simulink model "MX_Gateway"  (Simulink 9.0)
settings: Solver=FixedStepDiscrete  StartTime=0.0  StopTime=100  FixedStep=0.01  RelTol=1e-3
values from neighbouring files (symbols this model uses):
  ACTIVE = Bobcat.Parameter; CreateDefine( ACTIVE                         ,1                          ,'Define' ,'defines.h' )    [defines.m]
  ACTIVE_MODE_ONE = Bobcat.Parameter; CreateDefine( ACTIVE_MODE_ONE                ,19                         ,'Define' ,'defines.h' )    [defines.m]
  AUTHENTICATED = Bobcat.Parameter; CreateDefine( AUTHENTICATED                  ,1                          ,'Define' ,'defines.h' )    [defines.m]
  AUX_EMEA_CONTROLS = Bobcat.Parameter; CreateDefine( AUX_EMEA_CONTROLS  ,1   ,'Define' ,'defines.h' )    [enums.m]
  AUX_FLOW_HIGH = Bobcat.Parameter; CreateDefine( AUX_FLOW_HIGH           ,4  ,'Define' ,'defines.h' )    [enums.m]
  AUX_FLOW_INACTIVE = Bobcat.Parameter; CreateDefine( AUX_FLOW_INACTIVE       ,0  ,'Define' ,'defines.h' )    [enums.m]
  AUX_FLOW_LOW = Bobcat.Parameter; CreateDefine( AUX_FLOW_LOW            ,1  ,'Define' ,'defines.h' )    [enums.m]
  AUX_FLOW_MEDIUM = Bobcat.Parameter; CreateDefine( AUX_FLOW_MEDIUM         ,2  ,'Define' ,'defines.h' )    [enums.m]
  AUX_FLOW_STANDARD = Bobcat.Parameter; CreateDefine( AUX_FLOW_STANDARD       ,3  ,'Define' ,'defines.h' )    [enums.m]
  AUX_NON_FUNCTIONAL = Bobcat.Parameter; CreateDefine( AUX_NON_FUNCTIONAL ,255 ,'Define' ,'defines.h' )    [enums.m]
  BASE_ACTIVE = Bobcat.Parameter; CreateDefine( BASE_ACTIVE                    ,253                        ,'Define' ,'defines.h' )    [defines.m]
  BASE_DETENT = Bobcat.Parameter; CreateDefine( BASE_DETENT                    ,1                          ,'Define' ,'defines.h' )    [defines.m]
  BEEP_NONE = Bobcat.Parameter; CreateDefine( BEEP_NONE                      ,255                        ,'Define' ,'defines.h' )    [defines.m]
  CD_AIR_FILTER_DEBOUNCE_NORMAL = Bobcat.Parameter; CreateAliasCD( CD_AIR_FILTER_DEBOUNCE_NORMAL                        ,uint16(500)                ,'ConstantDataPointer' ,'CONSTANT_DATA' ,'AIR_FILTER_DEBOUNCE_NORMAL')    [cdata.m]    (also in hc12_cdata.m; m4_cdata.m)
  CD_AIR_FILTER_DEBOUNCE_NOT_CONNECTED = Bobcat.Parameter; CreateAliasCD( CD_AIR_FILTER_DEBOUNCE_NOT_CONNECTED                 ,uint16(3000)               ,'ConstantDataPointer' ,'CONSTANT_DATA' ,'AIR_FILTER_DEBOUNCE_NOT_CONNECTED')    [cdata.m]    (also in hc12_cdata.m; m4_cdata.m)
  CD_AIR_FILTER_DEBOUNCE_PLUGGED = Bobcat.Parameter; CreateAliasCD( CD_AIR_FILTER_DEBOUNCE_PLUGGED                       ,uint16(3000)               ,'ConstantDataPointer' ,'CONSTANT_DATA' ,'AIR_FILTER_DEBOUNCE_PLUGGED')    [cdata.m]    (also in hc12_cdata.m; m4_cdata.m)
  CD_AIR_FILTER_FUNCTION = Bobcat.Parameter; CreateAliasCD( CD_AIR_FILTER_FUNCTION                               ,uint8(255)                 ,'ConstantDataPointer' ,'CONSTANT_DATA' ,'AIR_FILTER_FUNCTION')    [cdata.m]    (also in hc12_cdata.m; m4_cdata.m)
  CD_AIR_FILTER_PLUGGED_WEIGHT = Bobcat.Parameter; CreateAliasCD( CD_AIR_FILTER_PLUGGED_WEIGHT                         ,uint8(2)                   ,'ConstantDataPointer' ,'CONSTANT_DATA' ,'AIR_FILTER_PLUGGED_WEIGHT')    [cdata.m]    (also in hc12_cdata.m; m4_cdata.m)
  CD_AIR_FILTER_UNPLUGGED_WEIGHT = Bobcat.Parameter; CreateAliasCD( CD_AIR_FILTER_UNPLUGGED_WEIGHT                       ,uint8(1)                   ,'ConstantDataPointer' ,'CONSTANT_DATA' ,'AIR_FILTER_UNPLUGGED_WEIGHT')    [cdata.m]    (also in hc12_cdata.m; m4_cdata.m)
  CD_AUTOIDLE_ARMBAR_DELAY = Bobcat.Parameter; CreateAliasCD( CD_AUTOIDLE_ARMBAR_DELAY                             ,uint8(2)                   ,'ConstantDataPointer' ,'CONSTANT_DATA' ,'AUTOIDLE_ARMBAR_DELAY')    [cdata.m]    (also in hc12_cdata.m; m4_cdata.m)
  CD_AUTOIDLE_FUNCTION = Bobcat.Parameter; CreateAliasCD( CD_AUTOIDLE_FUNCTION                                 ,uint8(255)                 ,'ConstantDataPointer' ,'CONSTANT_DATA' ,'AUTOIDLE_FUNCTION')    [cdata.m]    (also in hc12_cdata.m; m4_cdata.m)
  CD_AUTOIDLE_IDLE_DELAY = Bobcat.Parameter; CreateAliasCD( CD_AUTOIDLE_IDLE_DELAY                               ,uint8(40)                  ,'ConstantDataPointer' ,'CONSTANT_DATA' ,'AUTOIDLE_IDLE_DELAY')    [cdata.m]    (also in hc12_cdata.m; m4_cdata.m)
  CD_AUTOIDLE_LOW_IDLE_START = Bobcat.Parameter; CreateAliasCD( CD_AUTOIDLE_LOW_IDLE_START                           ,uint8(255)                 ,'ConstantDataPointer' ,'CONSTANT_DATA' ,'AUTOIDLE_LOW_IDLE_START')    [cdata.m]    (also in hc12_cdata.m; m4_cdata.m)
  CD_AUTOIDLE_PRESSURE_OFF = Bobcat.Parameter; CreateAliasCD( CD_AUTOIDLE_PRESSURE_OFF                             ,uint16(300)                ,'ConstantDataPointer' ,'CONSTANT_DATA' ,'AUTOIDLE_PRESSURE_OFF')    [cdata.m]    (also in hc12_cdata.m; m4_cdata.m)
  CD_AUTOIDLE_PRESSURE_ON = Bobcat.Parameter; CreateAliasCD( CD_AUTOIDLE_PRESSURE_ON                              ,uint16(270)                ,'ConstantDataPointer' ,'CONSTANT_DATA' ,'AUTOIDLE_PRESSURE_ON')    [cdata.m]    (also in hc12_cdata.m; m4_cdata.m)
  CD_AUTOSHIFT_FUNCTION = Bobcat.Parameter; CreateAliasCD( CD_AUTOSHIFT_FUNCTION                                ,uint8(255)                 ,'ConstantDataPointer' ,'CONSTANT_DATA' ,'AUTOSHIFT_FUNCTION')    [cdata.m]    (also in hc12_cdata.m; m4_cdata.m)
  CD_AUTOSHIFT_PHIGH1 = Bobcat.Parameter; CreateAliasCD( CD_AUTOSHIFT_PHIGH1                                  ,uint16(205)                ,'ConstantDataPointer' ,'CONSTANT_DATA' ,'AUTOSHIFT_PHIGH1')    [cdata.m]    (also in hc12_cdata.m; m4_cdata.m)
  CD_AUTOSHIFT_PHIGH2 = Bobcat.Parameter; CreateAliasCD( CD_AUTOSHIFT_PHIGH2                                  ,uint16(193)                ,'ConstantDataPointer' ,'CONSTANT_DATA' ,'AUTOSHIFT_PHIGH2')    [cdata.m]    (also in hc12_cdata.m; m4_cdata.m)
  CD_AUTOSHIFT_PHIGH_TEMP1 = Bobcat.Parameter; CreateAliasCD( CD_AUTOSHIFT_PHIGH_TEMP1                             ,int16(70)                  ,'ConstantDataPointer' ,'CONSTANT_DATA' ,'AUTOSHIFT_PHIGH_TEMP1')    [cdata.m]    (also in hc12_cdata.m; m4_cdata.m)
  CD_AUTOSHIFT_PHIGH_TEMP2 = Bobcat.Parameter; CreateAliasCD( CD_AUTOSHIFT_PHIGH_TEMP2                             ,int16(160)                 ,'ConstantDataPointer' ,'CONSTANT_DATA' ,'AUTOSHIFT_PHIGH_TEMP2')    [cdata.m]    (also in hc12_cdata.m; m4_cdata.m)
  CD_AUTOSHIFT_PLOW1 = Bobcat.Parameter; CreateAliasCD( CD_AUTOSHIFT_PLOW1                                   ,uint16(130)                ,'ConstantDataPointer' ,'CONSTANT_DATA' ,'AUTOSHIFT_PLOW1')    [cdata.m]    (also in hc12_cdata.m; m4_cdata.m)
  CD_AUTOSHIFT_PLOW2 = Bobcat.Parameter; CreateAliasCD( CD_AUTOSHIFT_PLOW2                                   ,uint16(100)                ,'ConstantDataPointer' ,'CONSTANT_DATA' ,'AUTOSHIFT_PLOW2')    [cdata.m]    (also in hc12_cdata.m; m4_cdata.m)
  CD_AUTOSHIFT_PLOW_TEMP1 = Bobcat.Parameter; CreateAliasCD( CD_AUTOSHIFT_PLOW_TEMP1                              ,int16(20)                  ,'ConstantDataPointer' ,'CONSTANT_DATA' ,'AUTOSHIFT_PLOW_TEMP1')    [cdata.m]    (also in hc12_cdata.m; m4_cdata.m)
  CD_AUTOSHIFT_PLOW_TEMP2 = Bobcat.Parameter; CreateAliasCD( CD_AUTOSHIFT_PLOW_TEMP2                              ,int16(80)                  ,'ConstantDataPointer' ,'CONSTANT_DATA' ,'AUTOSHIFT_PLOW_TEMP2')    [cdata.m]    (also in hc12_cdata.m; m4_cdata.m)
  CD_AUTOSHIFT_TRAVEL_DELAY = Bobcat.Parameter; CreateAliasCD( CD_AUTOSHIFT_TRAVEL_DELAY                            ,uint8(32)                  ,'ConstantDataPointer' ,'CONSTANT_DATA' ,'AUTOSHIFT_TRAVEL_DELAY')    [cdata.m]    (also in hc12_cdata.m; m4_cdata.m)
  CD_AUX2_CRACKPOINT_CURRENT = Bobcat.Parameter; CreateAliasCD( CD_AUX2_CRACKPOINT_CURRENT                           ,fi(0,0,16,12,'hex','0c28') ,'ConstantDataPointer' ,'CONSTANT_DATA' ,'AUX2_CRACKPOINT_CURRENT')    [cdata.m]    (also in hc12_cdata.m; m4_cdata.m)
  CD_AUX2_FLOW_LOW_FUNCTION = Bobcat.Parameter; CreateAliasCD( CD_AUX2_FLOW_LOW_FUNCTION                            ,uint8(255)                 ,'ConstantDataPointer' ,'CONSTANT_DATA' ,'AUX2_FLOW_LOW_FUNCTION')    [cdata.m]    (also in hc12_cdata.m; m4_cdata.m)
  CD_AUX2_FLOW_MED_FUNCTION = Bobcat.Parameter; CreateAliasCD( CD_AUX2_FLOW_MED_FUNCTION                            ,uint8(255)                 ,'ConstantDataPointer' ,'CONSTANT_DATA' ,'AUX2_FLOW_MED_FUNCTION')    [cdata.m]    (also in hc12_cdata.m; m4_cdata.m)
  CD_AUX2_HI_FLOW_PERCENT = Bobcat.Parameter; CreateAliasCD( CD_AUX2_HI_FLOW_PERCENT                              ,fi(0,0,16,16,'hex','ffff') ,'ConstantDataPointer' ,'CONSTANT_DATA' ,'AUX2_HI_FLOW_PERCENT')    [cdata.m]    (also in hc12_cdata.m; m4_cdata.m)
  CD_AUX2_LOW_FLOW_PERCENT = Bobcat.Parameter; CreateAliasCD( CD_AUX2_LOW_FLOW_PERCENT                             ,fi(0,0,16,16,'hex','7333') ,'ConstantDataPointer' ,'CONSTANT_DATA' ,'AUX2_LOW_FLOW_PERCENT')    [cdata.m]    (also in hc12_cdata.m; m4_cdata.m)
  CD_AUX2_MAX_FLOW_CURRENT = Bobcat.Parameter; CreateAliasCD( CD_AUX2_MAX_FLOW_CURRENT                             ,fi(0,0,16,12,'hex','14cc') ,'ConstantDataPointer' ,'CONSTANT_DATA' ,'AUX2_MAX_FLOW_CURRENT')    [cdata.m]    (also in hc12_cdata.m; m4_cdata.m)
  CD_AUX2_MED_FLOW_PERCENT = Bobcat.Parameter; CreateAliasCD( CD_AUX2_MED_FLOW_PERCENT                             ,fi(0,0,16,16,'hex','9999') ,'ConstantDataPointer' ,'CONSTANT_DATA' ,'AUX2_MED_FLOW_PERCENT')    [cdata.m]    (also in hc12_cdata.m; m4_cdata.m)
  CD_AUX_CRACKPOINT_CURRENT = Bobcat.Parameter; CreateAliasCD( CD_AUX_CRACKPOINT_CURRENT                            ,fi(0,0,16,12,'hex','0000') ,'ConstantDataPointer' ,'CONSTANT_DATA' ,'AUX_CRACKPOINT_CURRENT')    [cdata.m]    (also in hc12_cdata.m; m4_cdata.m)
  CD_AUX_DETENT_FLASH_TIME = Bobcat.Parameter; CreateAliasCD( CD_AUX_DETENT_FLASH_TIME                             ,uint16(72)                 ,'ConstantDataPointer' ,'CONSTANT_DATA' ,'AUX_DETENT_FLASH_TIME')    [cdata.m]    (also in hc12_cdata.m; m4_cdata.m)
  CD_AUX_DETENT_HOLD_TIME = Bobcat.Parameter; CreateAliasCD( CD_AUX_DETENT_HOLD_TIME                              ,uint16(100)                ,'ConstantDataPointer' ,'CONSTANT_DATA' ,'AUX_DETENT_HOLD_TIME')    [cdata.m]    (also in hc12_cdata.m; m4_cdata.m)
  CD_AUX_FULL_ON_AUX1 = Bobcat.Parameter; CreateAliasCD( CD_AUX_FULL_ON_AUX1                                  ,fi(0,0,16,12,'hex','10cd') ,'ConstantDataPointer' ,'CONSTANT_DATA' ,'AUX_FULL_ON_AUX1')    [cdata.m]    (also in hc12_cdata.m; m4_cdata.m)
  CD_AUX_FULL_ON_AUX2 = Bobcat.Parameter; CreateAliasCD( CD_AUX_FULL_ON_AUX2                                  ,fi(0,0,16,12,'hex','139d') ,'ConstantDataPointer' ,'CONSTANT_DATA' ,'AUX_FULL_ON_AUX2')    [cdata.m]    (also in hc12_cdata.m; m4_cdata.m)
  CD_AUX_FULL_ON_AUX3 = Bobcat.Parameter; CreateAliasCD( CD_AUX_FULL_ON_AUX3                                  ,fi(0,0,16,12,'hex','17d7') ,'ConstantDataPointer' ,'CONSTANT_DATA' ,'AUX_FULL_ON_AUX3')    [cdata.m]    (also in hc12_cdata.m; m4_cdata.m)
  CD_AUX_FUNCTION = Bobcat.Parameter; CreateAliasCD( CD_AUX_FUNCTION                                      ,uint8(0)                   ,'ConstantDataPointer' ,'CONSTANT_DATA' ,'AUX_FUNCTION')    [cdata.m]    (also in hc12_cdata.m; m4_cdata.m)
  CD_AUX_RATE_LIMIT_CRACKPOINT = Bobcat.Parameter; CreateAliasCD( CD_AUX_RATE_LIMIT_CRACKPOINT                         ,fi(0,0,16,12,'hex','0800') ,'ConstantDataPointer' ,'CONSTANT_DATA' ,'AUX_RATE_LIMIT_CRACKPOINT')    [cdata.m]    (also in hc12_cdata.m; m4_cdata.m)
  CD_AUX_RATE_LIMIT_CRACKPOINT_RATE = Bobcat.Parameter; CreateAliasCD( CD_AUX_RATE_LIMIT_CRACKPOINT_RATE                    ,fi(0,1,32,12,'int',102400) ,'ConstantDataPointer' ,'CONSTANT_DATA' ,'AUX_RATE_LIMIT_CRACKPOINT_RATE')    [cdata.m]    (also in hc12_cdata.m; m4_cdata.m)
  CD_AUX_RATE_LIMIT_FUNCTION = Bobcat.Parameter; CreateAliasCD( CD_AUX_RATE_LIMIT_FUNCTION                           ,uint8(255)                 ,'ConstantDataPointer' ,'CONSTANT_DATA' ,'AUX_RATE_LIMIT_FUNCTION')    [cdata.m]    (also in hc12_cdata.m; m4_cdata.m)
  CD_AUX_RATE_LIMIT_MAX_ENG_SPD = Bobcat.Parameter; CreateAliasCD( CD_AUX_RATE_LIMIT_MAX_ENG_SPD                        ,uint16(1600)               ,'ConstantDataPointer' ,'CONSTANT_DATA' ,'AUX_RATE_LIMIT_MAX_ENG_SPD')    [cdata.m]    (also in hc12_cdata.m; m4_cdata.m)
  CD_AUX_RATE_LIMIT_MIN_ENG_SPD = Bobcat.Parameter; CreateAliasCD( CD_AUX_RATE_LIMIT_MIN_ENG_SPD                        ,uint16(100)                ,'ConstantDataPointer' ,'CONSTANT_DATA' ,'AUX_RATE_LIMIT_MIN_ENG_SPD')    [cdata.m]    (also in hc12_cdata.m; m4_cdata.m)
  CD_AUX_RATE_LIMIT_NORMAL_RATE = Bobcat.Parameter; CreateAliasCD( CD_AUX_RATE_LIMIT_NORMAL_RATE                        ,fi(0,1,32,12,'int',2048)   ,'ConstantDataPointer' ,'CONSTANT_DATA' ,'AUX_RATE_LIMIT_NORMAL_RATE')    [cdata.m]    (also in hc12_cdata.m; m4_cdata.m)
  CD_AUXPOT_CAL_DEADBAND_HIGH = Bobcat.Parameter; CreateAliasCD( CD_AUXPOT_CAL_DEADBAND_HIGH                          ,uint16(574)                ,'ConstantDataPointer' ,'CONSTANT_DATA' ,'AUXPOT_CAL_DEADBAND_HIGH')    [cdata.m]    (also in hc12_cdata.m; m4_cdata.m)
  CD_AUXPOT_CAL_DEADBAND_LOW = Bobcat.Parameter; CreateAliasCD( CD_AUXPOT_CAL_DEADBAND_LOW                           ,uint16(424)                ,'ConstantDataPointer' ,'CONSTANT_DATA' ,'AUXPOT_CAL_DEADBAND_LOW')    [cdata.m]    (also in hc12_cdata.m; m4_cdata.m)
  CD_AUXPOT_CENTER_RATIO = Bobcat.Parameter; CreateAliasCD( CD_AUXPOT_CENTER_RATIO                               ,uint16(500)                ,'ConstantDataPointer' ,'CONSTANT_DATA' ,'AUXPOT_CENTER_RATIO')    [cdata.m]    (also in hc12_cdata.m; m4_cdata.m)
  CD_AUXPOT_DEADBAND = Bobcat.Parameter; CreateAliasCD( CD_AUXPOT_DEADBAND                                   ,uint8(38)                  ,'ConstantDataPointer' ,'CONSTANT_DATA' ,'AUXPOT_DEADBAND')    [cdata.m]    (also in hc12_cdata.m; m4_cdata.m)
  CD_AUXPOT_FUNCTION = Bobcat.Parameter; CreateAliasCD( CD_AUXPOT_FUNCTION                                   ,uint8(0)                   ,'ConstantDataPointer' ,'CONSTANT_DATA' ,'AUXPOT_FUNCTION')    [cdata.m]    (also in hc12_cdata.m; m4_cdata.m)
  CD_AUXPOT_RATIO_GAIN = Bobcat.Parameter; CreateAliasCD( CD_AUXPOT_RATIO_GAIN                                 ,uint16(1000)               ,'ConstantDataPointer' ,'CONSTANT_DATA' ,'AUXPOT_RATIO_GAIN')    [cdata.m]    (also in hc12_cdata.m; m4_cdata.m)
  CD_CAN_THROTTLE_FUNCTION = Bobcat.Parameter; CreateAliasCD( CD_CAN_THROTTLE_FUNCTION                             ,uint8(255)                 ,'ConstantDataPointer' ,'CONSTANT_DATA' ,'CAN_THROTTLE_FUNCTION')    [cdata.m]    (also in hc12_cdata.m; m4_cdata.m)
  CD_CAN_THROTTLE_REVERSE = Bobcat.Parameter; CreateAliasCD( CD_CAN_THROTTLE_REVERSE                              ,uint8(255)                 ,'ConstantDataPointer' ,'CONSTANT_DATA' ,'CAN_THROTTLE_REVERSE')    [cdata.m]    (also in hc12_cdata.m; m4_cdata.m)
  CD_CONSOLE_DEBOUNCE_ABSENT = Bobcat.Parameter; CreateAliasCD( CD_CONSOLE_DEBOUNCE_ABSENT                           ,uint16(12)                 ,'ConstantDataPointer' ,'CONSTANT_DATA' ,'CONSOLE_DEBOUNCE_ABSENT')    [cdata.m]    (also in hc12_cdata.m; m4_cdata.m)
  CD_CONSOLE_DEBOUNCE_OORH = Bobcat.Parameter; CreateAliasCD( CD_CONSOLE_DEBOUNCE_OORH                             ,uint16(12)                 ,'ConstantDataPointer' ,'CONSTANT_DATA' ,'CONSOLE_DEBOUNCE_OORH')    [cdata.m]    (also in hc12_cdata.m; m4_cdata.m)
  CD_CONSOLE_DEBOUNCE_OORL = Bobcat.Parameter; CreateAliasCD( CD_CONSOLE_DEBOUNCE_OORL                             ,uint16(12)                 ,'ConstantDataPointer' ,'CONSTANT_DATA' ,'CONSOLE_DEBOUNCE_OORL')    [cdata.m]    (also in hc12_cdata.m; m4_cdata.m)
  CD_CONSOLE_DEBOUNCE_PRESENT = Bobcat.Parameter; CreateAliasCD( CD_CONSOLE_DEBOUNCE_PRESENT                          ,uint16(12)                 ,'ConstantDataPointer' ,'CONSTANT_DATA' ,'CONSOLE_DEBOUNCE_PRESENT')    [cdata.m]    (also in hc12_cdata.m; m4_cdata.m)
  CD_CONSOLE_FUNCTION = Bobcat.Parameter; CreateAliasCD( CD_CONSOLE_FUNCTION                                  ,uint8(0)                   ,'ConstantDataPointer' ,'CONSTANT_DATA' ,'CONSOLE_FUNCTION')    [cdata.m]    (also in hc12_cdata.m; m4_cdata.m)
  CD_CONSOLE_OORH = Bobcat.Parameter; CreateAliasCD( CD_CONSOLE_OORH                                      ,uint16(740)                ,'ConstantDataPointer' ,'CONSTANT_DATA' ,'CONSOLE_OORH')    [cdata.m]    (also in hc12_cdata.m; m4_cdata.m)
  CD_CONSOLE_OORL = Bobcat.Parameter; CreateAliasCD( CD_CONSOLE_OORL                                      ,uint16(10)                 ,'ConstantDataPointer' ,'CONSTANT_DATA' ,'CONSOLE_OORL')    [cdata.m]    (also in hc12_cdata.m; m4_cdata.m)
  CD_CONSOLE_PRESENT_HIGH = Bobcat.Parameter; CreateAliasCD( CD_CONSOLE_PRESENT_HIGH                              ,uint16(515)                ,'ConstantDataPointer' ,'CONSTANT_DATA' ,'CONSOLE_PRESENT_HIGH')    [cdata.m]    (also in hc12_cdata.m; m4_cdata.m)
  CD_CONSOLE_PRESENT_LOW = Bobcat.Parameter; CreateAliasCD( CD_CONSOLE_PRESENT_LOW                               ,uint16(375)                ,'ConstantDataPointer' ,'CONSTANT_DATA' ,'CONSOLE_PRESENT_LOW')    [cdata.m]    (also in hc12_cdata.m; m4_cdata.m)
  CD_DELUXE_GEN5_5_FUNCTION = Bobcat.Parameter; CreateAliasCD( CD_DELUXE_GEN5_5_FUNCTION                            ,uint8(255)                 ,'ConstantDataPointer' ,'CONSTANT_DATA' ,'DELUXE_GEN5_5_FUNCTION')    [cdata.m]    (also in hc12_cdata.m; m4_cdata.m)
  CD_DELUXE_GEN5_FUNCTION = Bobcat.Parameter; CreateAliasCD( CD_DELUXE_GEN5_FUNCTION                              ,uint8(255)                 ,'ConstantDataPointer' ,'CONSTANT_DATA' ,'DELUXE_GEN5_FUNCTION')    [cdata.m]    (also in hc12_cdata.m; m4_cdata.m)
  CD_DERATE_ENGINE_SPEED_DEBOUNCE_HIGH = Bobcat.Parameter; CreateAliasCD( CD_DERATE_ENGINE_SPEED_DEBOUNCE_HIGH                 ,uint16(200)                ,'ConstantDataPointer' ,'CONSTANT_DATA' ,'DERATE_ENGINE_SPEED_DEBOUNCE_HIGH')    [cdata.m]    (also in hc12_cdata.m; m4_cdata.m)
  CD_DERATE_ENGINE_SPEED_DEBOUNCE_SHUTDOWN = Bobcat.Parameter; CreateAliasCD( CD_DERATE_ENGINE_SPEED_DEBOUNCE_SHUTDOWN             ,uint16(1000)               ,'ConstantDataPointer' ,'CONSTANT_DATA' ,'DERATE_ENGINE_SPEED_DEBOUNCE_SHUTDOWN')    [cdata.m]    (also in hc12_cdata.m; m4_cdata.m)
  CD_DERATE_ENGINE_SPEED_HIGH_OFFSET = Bobcat.Parameter; CreateAliasCD( CD_DERATE_ENGINE_SPEED_HIGH_OFFSET                   ,uint16(200)                ,'ConstantDataPointer' ,'CONSTANT_DATA' ,'DERATE_ENGINE_SPEED_HIGH_OFFSET')    [cdata.m]    (also in hc12_cdata.m; m4_cdata.m)
  CD_DERATE_METHOD = Bobcat.Parameter; CreateAliasCD( CD_DERATE_METHOD                                     ,uint8(0)                   ,'ConstantDataPointer' ,'CONSTANT_DATA' ,'DERATE_METHOD')    [cdata.m]    (also in hc12_cdata.m; m4_cdata.m)
  CD_DUMP_VALVE_FUNCTION = Bobcat.Parameter; CreateAliasCD( CD_DUMP_VALVE_FUNCTION                               ,uint8(255)                 ,'ConstantDataPointer' ,'CONSTANT_DATA' ,'DUMP_VALVE_FUNCTION')    [cdata.m]    (also in hc12_cdata.m; m4_cdata.m)
  CD_DUMP_VALVE_ON_CURRENT = Bobcat.Parameter; CreateAliasCD( CD_DUMP_VALVE_ON_CURRENT                             ,fi(0,0,16,12,'hex','ffff') ,'ConstantDataPointer' ,'CONSTANT_DATA' ,'DUMP_VALVE_ON_CURRENT')    [cdata.m]    (also in hc12_cdata.m; m4_cdata.m)
  CD_ECO_MODE_THROTTLE_REDUCTION = Bobcat.Parameter; CreateAliasCD( CD_ECO_MODE_THROTTLE_REDUCTION                       ,uint16(850)                ,'ConstantDataPointer' ,'CONSTANT_DATA' ,'ECO_MODE_THROTTLE_REDUCTION')    [cdata.m]    (also in hc12_cdata.m; m4_cdata.m)
  CD_ECT_FILTER_GAIN = Bobcat.Parameter; CreateAliasCD( CD_ECT_FILTER_GAIN                                   ,uint8(6)                   ,'ConstantDataPointer' ,'CONSTANT_DATA' ,'ECT_FILTER_GAIN')    [cdata.m]    (also in hc12_cdata.m; m4_cdata.m)
  CD_ECU_FUNCTION = Bobcat.Parameter; CreateAliasCD( CD_ECU_FUNCTION                                      ,uint8(255)                 ,'ConstantDataPointer' ,'CONSTANT_DATA' ,'ECU_FUNCTION')    [cdata.m]    (also in hc12_cdata.m; m4_cdata.m)
  CD_ECU_HOLD_DEBOUNCE_ERROR_ON = Bobcat.Parameter; CreateAliasCD( CD_ECU_HOLD_DEBOUNCE_ERROR_ON                        ,uint16(20)                 ,'ConstantDataPointer' ,'CONSTANT_DATA' ,'ECU_HOLD_DEBOUNCE_ERROR_ON')    [cdata.m]    (also in hc12_cdata.m; m4_cdata.m)
  CD_EMEA_CONTROLS_HOLD_TIME = Bobcat.Parameter; CreateAliasCD( CD_EMEA_CONTROLS_HOLD_TIME                           ,uint16(30)                 ,'ConstantDataPointer' ,'CONSTANT_DATA' ,'EMEA_CONTROLS_HOLD_TIME')    [cdata.m]    (also in hc12_cdata.m; m4_cdata.m)
  CD_EMEA_CONTROLS_OPTION_A = Bobcat.Parameter; CreateAliasCD( CD_EMEA_CONTROLS_OPTION_A                            ,uint8(255)                 ,'ConstantDataPointer' ,'CONSTANT_DATA' ,'EMEA_CONTROLS_OPTION_A')    [cdata.m]    (also in hc12_cdata.m; m4_cdata.m)
  CD_EMEA_CONTROLS_OPTION_C = Bobcat.Parameter; CreateAliasCD( CD_EMEA_CONTROLS_OPTION_C                            ,uint8(255)                 ,'ConstantDataPointer' ,'CONSTANT_DATA' ,'EMEA_CONTROLS_OPTION_C')    [cdata.m]    (also in hc12_cdata.m; m4_cdata.m)
  CD_EMEA_CONTROLS_REVERSE = Bobcat.Parameter; CreateAliasCD( CD_EMEA_CONTROLS_REVERSE                             ,uint8(255)                 ,'ConstantDataPointer' ,'CONSTANT_DATA' ,'EMEA_CONTROLS_REVERSE')    [cdata.m]    (also in hc12_cdata.m; m4_cdata.m)
  CD_EMEA_ELECTRIC_VERSION = Bobcat.Parameter; CreateAliasCD( CD_EMEA_ELECTRIC_VERSION                             ,uint8(0)                   ,'ConstantDataPointer' ,'CONSTANT_DATA' ,'EMEA_ELECTRIC_VERSION')    [cdata.m]    (also in hc12_cdata.m; m4_cdata.m)
  CD_EMEA_POT_CAL_DEADBAND_HIGH = Bobcat.Parameter; CreateAliasCD( CD_EMEA_POT_CAL_DEADBAND_HIGH                        ,uint16(574)                ,'ConstantDataPointer' ,'CONSTANT_DATA' ,'EMEA_POT_CAL_DEADBAND_HIGH')    [cdata.m]    (also in hc12_cdata.m; m4_cdata.m)
  CD_EMEA_POT_CAL_DEADBAND_LOW = Bobcat.Parameter; CreateAliasCD( CD_EMEA_POT_CAL_DEADBAND_LOW                         ,uint16(424)                ,'ConstantDataPointer' ,'CONSTANT_DATA' ,'EMEA_POT_CAL_DEADBAND_LOW')    [cdata.m]    (also in hc12_cdata.m; m4_cdata.m)
  CD_ENGINE_RPM_FACTOR = Bobcat.Parameter; CreateAliasCD( CD_ENGINE_RPM_FACTOR                                 ,uint8(35)                  ,'ConstantDataPointer' ,'CONSTANT_DATA' ,'ENGINE_RPM_FACTOR')    [cdata.m]    (also in hc12_cdata.m; m4_cdata.m)
  CD_ENGINE_SPEED_FILTER_FAST_VALUE = Bobcat.Parameter; CreateAliasCD( CD_ENGINE_SPEED_FILTER_FAST_VALUE                    ,uint8(1)                   ,'ConstantDataPointer' ,'CONSTANT_DATA' ,'ENGINE_SPEED_FILTER_FAST_VALUE')    [cdata.m]    (also in hc12_cdata.m; m4_cdata.m)
  CD_ENGINE_SPEED_FILTER_SLOW_VALUE = Bobcat.Parameter; CreateAliasCD( CD_ENGINE_SPEED_FILTER_SLOW_VALUE                    ,uint8(4)                   ,'ConstantDataPointer' ,'CONSTANT_DATA' ,'ENGINE_SPEED_FILTER_SLOW_VALUE')    [cdata.m]    (also in hc12_cdata.m; m4_cdata.m)
  CD_ENGINE_SPEED_RUNNING = Bobcat.Parameter; CreateAliasCD( CD_ENGINE_SPEED_RUNNING                              ,uint16(800)                ,'ConstantDataPointer' ,'CONSTANT_DATA' ,'ENGINE_SPEED_RUNNING')    [cdata.m]    (also in hc12_cdata.m; m4_cdata.m)
  CD_ENGINE_START_DELAY_DEBOUNCE_RPM_NO_SIGNAL = Bobcat.Parameter; CreateAliasCD( CD_ENGINE_START_DELAY_DEBOUNCE_RPM_NO_SIGNAL         ,uint16(0)                  ,'ConstantDataPointer' ,'CONSTANT_DATA' ,'ENGINE_START_DELAY_DEBOUNCE_RPM_NO_SIGNAL')    [cdata.m]    (also in hc12_cdata.m; m4_cdata.m)
  CD_ENGINE_START_DELAY_OFF_TIME = Bobcat.Parameter; CreateAliasCD( CD_ENGINE_START_DELAY_OFF_TIME                       ,uint16(0)                  ,'ConstantDataPointer' ,'CONSTANT_DATA' ,'ENGINE_START_DELAY_OFF_TIME')    [cdata.m]    (also in hc12_cdata.m; m4_cdata.m)
  CD_ENGINE_START_DELAY_RPM_NO_SIGNAL = Bobcat.Parameter; CreateAliasCD( CD_ENGINE_START_DELAY_RPM_NO_SIGNAL                  ,uint16(0)                  ,'ConstantDataPointer' ,'CONSTANT_DATA' ,'ENGINE_START_DELAY_RPM_NO_SIGNAL')    [cdata.m]    (also in hc12_cdata.m; m4_cdata.m)
  CD_EOP_DEBOUNCE_EXTREMELY_LOW = Bobcat.Parameter; CreateAliasCD( CD_EOP_DEBOUNCE_EXTREMELY_LOW                        ,uint16(50)                 ,'ConstantDataPointer' ,'CONSTANT_DATA' ,'EOP_DEBOUNCE_EXTREMELY_LOW')    [cdata.m]    (also in hc12_cdata.m; m4_cdata.m)
  CD_EOP_DEBOUNCE_NORMAL = Bobcat.Parameter; CreateAliasCD( CD_EOP_DEBOUNCE_NORMAL                               ,uint16(50)                 ,'ConstantDataPointer' ,'CONSTANT_DATA' ,'EOP_DEBOUNCE_NORMAL')    [cdata.m]    (also in hc12_cdata.m; m4_cdata.m)
  CD_EOP_DEBOUNCE_SHUTDOWN = Bobcat.Parameter; CreateAliasCD( CD_EOP_DEBOUNCE_SHUTDOWN                             ,uint16(100)                ,'ConstantDataPointer' ,'CONSTANT_DATA' ,'EOP_DEBOUNCE_SHUTDOWN')    [cdata.m]    (also in hc12_cdata.m; m4_cdata.m)
  CD_EOP_FILTER_VALUE = Bobcat.Parameter; CreateAliasCD( CD_EOP_FILTER_VALUE                                  ,uint8(3)                   ,'ConstantDataPointer' ,'CONSTANT_DATA' ,'EOP_FILTER_VALUE')    [cdata.m]    (also in hc12_cdata.m; m4_cdata.m)
  CD_EOP_FUNCTION = Bobcat.Parameter; CreateAliasCD( CD_EOP_FUNCTION                                      ,uint8(1)                   ,'ConstantDataPointer' ,'CONSTANT_DATA' ,'EOP_FUNCTION')    [cdata.m]    (also in hc12_cdata.m; m4_cdata.m)
  CD_EOP_SWITCH_GAUGE_VALUE = Bobcat.Parameter; CreateAliasCD( CD_EOP_SWITCH_GAUGE_VALUE                            ,uint16(125)                ,'ConstantDataPointer' ,'CONSTANT_DATA' ,'EOP_SWITCH_GAUGE_VALUE')    [cdata.m]    (also in hc12_cdata.m; m4_cdata.m)
  CD_EOP_SWITCH_LOW = Bobcat.Parameter; CreateAliasCD( CD_EOP_SWITCH_LOW                                    ,uint16(50)                 ,'ConstantDataPointer' ,'CONSTANT_DATA' ,'EOP_SWITCH_LOW')    [cdata.m]    (also in hc12_cdata.m; m4_cdata.m)
  CD_EOP_SWITCH_PRESSURE_VALUE = Bobcat.Parameter; CreateAliasCD( CD_EOP_SWITCH_PRESSURE_VALUE                         ,uint16(40)                 ,'ConstantDataPointer' ,'CONSTANT_DATA' ,'EOP_SWITCH_PRESSURE_VALUE')    [cdata.m]    (also in hc12_cdata.m; m4_cdata.m)
  CD_EOT_DEBOUNCE_EXTREMELY_LOW = Bobcat.Parameter; CreateAliasCD( CD_EOT_DEBOUNCE_EXTREMELY_LOW                        ,uint16(2)                  ,'ConstantDataPointer' ,'CONSTANT_DATA' ,'EOT_DEBOUNCE_EXTREMELY_LOW')    [cdata.m]    (also in hc12_cdata.m; m4_cdata.m)
  CD_EOT_DEBOUNCE_LOW = Bobcat.Parameter; CreateAliasCD( CD_EOT_DEBOUNCE_LOW                                  ,uint16(2)                  ,'ConstantDataPointer' ,'CONSTANT_DATA' ,'EOT_DEBOUNCE_LOW')    [cdata.m]    (also in hc12_cdata.m; m4_cdata.m)
  CD_EOT_DEBOUNCE_NORMAL = Bobcat.Parameter; CreateAliasCD( CD_EOT_DEBOUNCE_NORMAL                               ,uint16(2)                  ,'ConstantDataPointer' ,'CONSTANT_DATA' ,'EOT_DEBOUNCE_NORMAL')    [cdata.m]    (also in hc12_cdata.m; m4_cdata.m)
  CD_EOT_EXTREMELY_LOW = Bobcat.Parameter; CreateAliasCD( CD_EOT_EXTREMELY_LOW                                 ,uint16(81)                 ,'ConstantDataPointer' ,'CONSTANT_DATA' ,'EOT_EXTREMELY_LOW')    [cdata.m]    (also in hc12_cdata.m; m4_cdata.m)
  CD_EOT_FUNCTION = Bobcat.Parameter; CreateAliasCD( CD_EOT_FUNCTION                                      ,uint8(255)                 ,'ConstantDataPointer' ,'CONSTANT_DATA' ,'EOT_FUNCTION')    [cdata.m]    (also in hc12_cdata.m; m4_cdata.m)
  CD_EOT_LOW = Bobcat.Parameter; CreateAliasCD( CD_EOT_LOW                                           ,uint16(90)                 ,'ConstantDataPointer' ,'CONSTANT_DATA' ,'EOT_LOW')    [cdata.m]    (also in hc12_cdata.m; m4_cdata.m)
  CD_EOT_TEMPERATURE_HYSTERESIS = Bobcat.Parameter; CreateAliasCD( CD_EOT_TEMPERATURE_HYSTERESIS                        ,uint16(2)                  ,'ConstantDataPointer' ,'CONSTANT_DATA' ,'EOT_TEMPERATURE_HYSTERESIS')    [cdata.m]    (also in hc12_cdata.m; m4_cdata.m)
  CD_FAST_TRAVEL_FUNCTION = Bobcat.Parameter; CreateAliasCD( CD_FAST_TRAVEL_FUNCTION                              ,uint8(255)                 ,'ConstantDataPointer' ,'CONSTANT_DATA' ,'FAST_TRAVEL_FUNCTION')    [cdata.m]    (also in hc12_cdata.m; m4_cdata.m)
  CD_FAST_TRAVEL_THROTTLE_DELAY = Bobcat.Parameter; CreateAliasCD( CD_FAST_TRAVEL_THROTTLE_DELAY                        ,uint16(10)                 ,'ConstantDataPointer' ,'CONSTANT_DATA' ,'FAST_TRAVEL_THROTTLE_DELAY')    [cdata.m]    (also in hc12_cdata.m; m4_cdata.m)
  CD_FAST_TRAVEL_THROTTLE_LIMIT = Bobcat.Parameter; CreateAliasCD( CD_FAST_TRAVEL_THROTTLE_LIMIT                        ,uint16(1000)               ,'ConstantDataPointer' ,'CONSTANT_DATA' ,'FAST_TRAVEL_THROTTLE_LIMIT')    [cdata.m]    (also in hc12_cdata.m; m4_cdata.m)
  CD_FLYWHEEL_TEETH = Bobcat.Parameter; CreateAliasCD( CD_FLYWHEEL_TEETH                                    ,uint8(83)                  ,'ConstantDataPointer' ,'CONSTANT_DATA' ,'FLYWHEEL_TEETH')    [cdata.m]    (also in hc12_cdata.m; m4_cdata.m)
  CD_FUEL_LEVEL_FILTER_FAST_VALUE = Bobcat.Parameter; CreateAliasCD( CD_FUEL_LEVEL_FILTER_FAST_VALUE                      ,uint8(5)                   ,'ConstantDataPointer' ,'CONSTANT_DATA' ,'FUEL_LEVEL_FILTER_FAST_VALUE')    [cdata.m]    (also in hc12_cdata.m; m4_cdata.m)
  CD_FUEL_LEVEL_FILTER_SLOW_VALUE = Bobcat.Parameter; CreateAliasCD( CD_FUEL_LEVEL_FILTER_SLOW_VALUE                      ,uint8(10)                  ,'ConstantDataPointer' ,'CONSTANT_DATA' ,'FUEL_LEVEL_FILTER_SLOW_VALUE')    [cdata.m]    (also in hc12_cdata.m; m4_cdata.m)
  CD_FUEL_PRIMARY_FEEDBACK_FUNCTION = Bobcat.Parameter; CreateAliasCD( CD_FUEL_PRIMARY_FEEDBACK_FUNCTION                    ,uint8(0)                   ,'ConstantDataPointer' ,'CONSTANT_DATA' ,'FUEL_PRIMARY_FEEDBACK_FUNCTION')    [cdata.m]    (also in hc12_cdata.m; m4_cdata.m)
  ... (+246 more)
undefined symbols (not defined in the file or in its folder): sigbuilder_block
undefined symbols (not defined in the file): CD_FUEL_RESOLUTION_TIMER
libraries: simulink x186, MX_Library x107
(part 40 of 48)

== stateflow chart MX_Gtwy_Control/System/Keyless_Entry/Power_Hold ==
  note: can_KeylessEntry[] initialized to 0xFF, so when all are 0, we know it is awake
      | and that no buttons are being pressed after the initial press to power it up.
  state NON_FUNCTIONAL
    en:
    state_KeylessEntryPowerHold = KE_PWR_INACTIVE;
  state WAIT_FOR_NO_PRESS
    en:
    state_KeylessEntryPowerHold = KE_PWR_ACTIVE;
  state KEYLESS_ENTRY_POWER_OFF
    en:
    state_KeylessEntryPowerHold = KE_PWR_INACTIVE;
  state WAIT_FOR_KEYLESS_ENTRY
  state KEYLESS_ENTRY_DETECTED
  state KEYLESS_ENTRY_VALID
  (default) -> NON_FUNCTIONAL
  NON_FUNCTIONAL -> WAIT_FOR_NO_PRESS : [CD_KEYLESS_ENTRY_FUNCTION != NON_FUNCTIONAL]
  WAIT_FOR_NO_PRESS -> KEYLESS_ENTRY_POWER_OFF [order 1] : %Error condition - no CANand/or Sw Pwr to keypad
      | [after(100, tick) || cabKeyDetect]
  WAIT_FOR_NO_PRESS -> WAIT_FOR_KEYLESS_ENTRY [order 2] : [(can_KeylessEntry[0] == 0) && (can_KeylessEntry[1] == 0) && (can_KeylessEntry[2] == 0)]
  WAIT_FOR_KEYLESS_ENTRY -> KEYLESS_ENTRY_POWER_OFF [order 2] : %No keypad press after powerup or machine key on
      | [after(CD_KEYLESS_ENTRY_WAIT_TIMEOUT,tick)...
      | ||cabKeyDetect]
  WAIT_FOR_KEYLESS_ENTRY -> KEYLESS_ENTRY_DETECTED [order 1] : [(can_KeylessEntry[0] != 0) || (can_KeylessEntry[1] != 0) || (can_KeylessEntry[2] != 0)]
  KEYLESS_ENTRY_DETECTED -> KEYLESS_ENTRY_DETECTED [order 1] : [(can_KeylessEntry[0] != 0) || (can_KeylessEntry[1] != 0) || (can_KeylessEntry[2] != 0)]
  KEYLESS_ENTRY_DETECTED -> KEYLESS_ENTRY_POWER_OFF [order 2] : %Timeout after keypad entry started or machine key on
      | [after(CD_KEYLESS_ENTRY_DETECTED_TIMEOUT,tick)...
      | ||cabKeyDetect]
  KEYLESS_ENTRY_DETECTED -> KEYLESS_ENTRY_VALID [order 3] : [state_KeylessEntryPassword == KE_PASS]
  KEYLESS_ENTRY_VALID -> KEYLESS_ENTRY_VALID [order 1] : [(can_KeylessEntry[0] != 0) || (can_KeylessEntry[1] != 0) || (can_KeylessEntry[2] != 0)]
  KEYLESS_ENTRY_VALID -> KEYLESS_ENTRY_POWER_OFF [order 2] : %Timeout after valid code entered or machine key on
      | %might need different timeout locked versus unlocked
      | [after(CD_KEYLESS_ENTRY_VALID_TIMEOUT,tick)...
      | || cabKeyDetect]
  data: state_KeylessEntryPowerHold output uint8; KE_PWR_INACTIVE parameter uint8; KE_PWR_ACTIVE parameter uint8; can_KeylessEntry input uint8 size=3; KE_PASS parameter uint8; CD_KEYLESS_ENTRY_WAIT_TIMEOUT parameter uint16; CD_KEYLESS_ENTRY_DETECTED_TIMEOUT parameter uint16; CD_KEYLESS_ENTRY_VALID_TIMEOUT parameter uint16; CD_KEYLESS_ENTRY_FUNCTION parameter uint8; NON_FUNCTIONAL parameter uint8; cabKeyDetect input boolean; state_KeylessEntryPassword input uint8

== stateflow chart MX_Gtwy_Control/System/Keyless_Entry/Indicators ==
  state NORMAL
    state BOTH_OFF
      en:
      ind_KeylessEntryLock = INACTIVE;
      ind_KeylessEntryUnlock = INACTIVE;
    state LOCK
      en:
      ind_KeylessEntryLock = ACTIVE;
      ind_KeylessEntryUnlock = INACTIVE;
    state UNLOCK
      en:
      ind_KeylessEntryLock = INACTIVE;
      ind_KeylessEntryUnlock = ACTIVE;
    (default) -> BOTH_OFF
    BOTH_OFF -> UNLOCK [order 2] : [state_KeylessEntryLock == KE_UNLOCK]
    BOTH_OFF -> LOCK [order 1] : [state_KeylessEntryLock == KE_LOCK]
    LOCK -> UNLOCK : [state_KeylessEntryLock == KE_UNLOCK]
    UNLOCK -> LOCK : [state_KeylessEntryLock == KE_LOCK]
  state FAIL
    state FLASHING
      state ACTIVE
        en:
        ind_KeylessEntryLock = ACTIVE;
        ind_KeylessEntryUnlock = ACTIVE;
      state INACTIVE
        en:
        ind_KeylessEntryLock = INACTIVE;
        ind_KeylessEntryUnlock = INACTIVE;
      (default) -> ACTIVE
      ACTIVE -> INACTIVE : [after(CD_KEYLESS_ENTRY_FLASH_RATE,tick)]
      INACTIVE -> ACTIVE : [after(CD_KEYLESS_ENTRY_FLASH_RATE, tick)]
    state TIMEOUT
      en:
      ind_KeylessEntryLock = INACTIVE;
      ind_KeylessEntryUnlock = INACTIVE;
    (default) -> FLASHING
    FLASHING -> TIMEOUT : [after(CD_KEYLESS_ENTRY_DETECTED_TIMEOUT,tick)]
  (default) -> NORMAL
  NORMAL -> FAIL : [state_KeylessEntryPassword == KE_FAIL]
  FAIL -> NORMAL : [state_KeylessEntryPassword != KE_FAIL]
  data: state_KeylessEntryPassword input uint8; ind_KeylessEntryLock output boolean; KE_UNLOCK parameter uint8; state_KeylessEntryLock input uint8; KE_LOCK parameter uint8; CD_KEYLESS_ENTRY_FLASH_RATE parameter uint16; ind_KeylessEntryUnlock output boolean; KE_FAIL parameter uint8; INACTIVE parameter uint8; ACTIVE parameter uint8; CD_KEYLESS_ENTRY_DETECTED_TIMEOUT parameter uint16

== stateflow chart MX_Gtwy_Control/Inputs/Offset_Pot/CAN_Pot/Pot ==
  state Pot_Calibration [parallel]
    settings: executionOrder=1
    state InitDelay
      en:
      state_OffsetPotCal = INACTIVE;
      offset_OffsetPotCal = 0;
    state Wait_For_Cal_Attempt
    state Wait_For_New_Cal
    state Joystick_Powerup
    (default) -> InitDelay
    InitDelay -> Wait_For_Cal_Attempt : [after(ONE_SECOND,tick)]
    Wait_For_Cal_Attempt -> (junction 4454) : [state_Console == ACTIVE...
        |  && state_Engine != ENGINE_CRANKING...
        |  && msgAge_BJM2Left < 100]
    (junction 4454) -> (junction 4455) [order 1] : [rm_OffsetPot >= CD_OFFSET_POT_CAL_DEADBAND_LOW...
        | && rm_OffsetPot <= CD_OFFSET_POT_CAL_DEADBAND_HIGH]
    (junction 4454) -> (junction 4457) [order 2] : {
        | state_OffsetPotCal = NOT_IN_NEUTRAL;
        | offset_OffsetPotCal = 0;
        | state_Buzzer = TRIPLE_SHORT_BEEP;
        | }
    (junction 4455) -> (junction 4456) : {
        | state_OffsetPotCal = NORMAL;
        | offset_OffsetPotCal = int16(CD_OFFSET_POT_CENTER_RATIO) - rm_OffsetPot;
        | }
    (junction 4456) -> Wait_For_New_Cal
    (junction 4457) -> Wait_For_New_Cal
    Wait_For_New_Cal -> Wait_For_Cal_Attempt [order 1] : [state_Console != ACTIVE]
    Wait_For_New_Cal -> Joystick_Powerup [order 2] : [state_Engine == ENGINE_CRANKING]
    Joystick_Powerup -> Wait_For_Cal_Attempt : [after(ONE_SECOND,tick)]
  state Pot_State [parallel]
    settings: executionOrder=2
    state CAN_OK
      state NORMAL
        state Inactive
          en:
          state_OffsetPot = NEUTRAL;
          base_Threshold = CD_OFFSET_POT_CENTER_RATIO + CD_OFFSET_POT_DEADBAND;
          rod_Threshold = CD_OFFSET_POT_CENTER_RATIO - CD_OFFSET_POT_DEADBAND;
          position_OffsetPotOut = CD_OFFSET_POT_CENTER_RATIO;
        state ACTIVE
          en:
          position_OffsetPotIn = rm_OffsetPot + offset_OffsetPotCal;
          position_OffsetPotOut = position_OffsetPotIn;
          du:
          position_OffsetPotIn = rm_OffsetPot + offset_OffsetPotCal;
          position_OffsetPotOut = position_OffsetPotIn;
          state Inhibit
            en:
            state_OffsetPot = NEUTRAL;
          state Normal
            state Neutral
              en:
              state_OffsetPot = NEUTRAL;
            state Base_Active
              en:
              state_OffsetPot =BASE_ACTIVE;
            state Rod_Active
              en:
              state_OffsetPot = ROD_ACTIVE;
            (default) -> Neutral
            Neutral -> Base_Active [order 2] : [position_OffsetPotIn > base_Threshold]
            Neutral -> Rod_Active [order 1] : [position_OffsetPotIn < rod_Threshold]
            Base_Active -> Neutral [order 2] : [position_OffsetPotIn <= base_Threshold]
            Rod_Active -> Neutral [order 2] : [position_OffsetPotIn >= rod_Threshold]
            Rod_Active -> Base_Active [order 1] : [position_OffsetPotIn >= rod_Threshold...
                |  && position_OffsetPotIn > base_Threshold]
            Base_Active -> Rod_Active [order 1] : [position_OffsetPotIn <= base_Threshold...
                |  && position_OffsetPotIn < rod_Threshold]
          (default) -> Inhibit
          Inhibit -> Normal : [state_JoystickEnable == ACTIVE...
              |  && position_OffsetPotIn <= base_Threshold...
              |  && position_OffsetPotIn >= rod_Threshold]
          Normal -> Inhibit : [state_JoystickEnable != ACTIVE]
        (default) -> Inactive
        Inactive -> ACTIVE : [state_OffsetPotCal != INACTIVE]
      state NOT_IN_NEUTRAL
        en:
        state_OffsetPot = NOT_IN_NEUTRAL;
        position_OffsetPotOut =...
        CD_OFFSET_POT_CENTER_RATIO;
      (default) -> NORMAL
      NORMAL -> NOT_IN_NEUTRAL : [state_OffsetPotCal == NOT_IN_NEUTRAL]
      NOT_IN_NEUTRAL -> NORMAL : [state_OffsetPotCal != NOT_IN_NEUTRAL]
    state CANFault
      en:
      state_OffsetPot = NORMAL;
      position_OffsetPotOut =...
      CD_OFFSET_POT_CENTER_RATIO;
    (default) -> CAN_OK
    CAN_OK -> CANFault : [msgAge_BJM2Left > 1000]
  data: rm_OffsetPot input int16; msgAge_BJM2Left input uint16; state_OffsetPot output uint8; position_OffsetPotOut output int16; position_OffsetPotIn local int16 size=1; offset_OffsetPotCal local int16; state_OffsetPotCal local uint8; CD_OFFSET_POT_CENTER_RATIO parameter uint16; CD_OFFSET_POT_CAL_DEADBAND_HIGH parameter uint16; CD_OFFSET_POT_CAL_DEADBAND_LOW parameter uint16; NORMAL parameter uint8; NOT_IN_NEUTRAL parameter uint8; BASE_ACTIVE parameter uint8; base_Threshold local uint16; CD_OFFSET_POT_DEADBAND parameter uint8; NEUTRAL parameter uint8; ROD_ACTIVE parameter uint8; rod_Threshold local uint16; ENGINE_CRANKING parameter uint8; state_Console input uint8; state_Engine input uint8; ONE_SECOND constant uint8 =100; INACTIVE parameter uint8; state_JoystickEnable input uint8; ACTIVE parameter uint8; TRIPLE_SHORT_BEEP parameter uint8; state_Buzzer data_store_memory double

== matlab function MX_Gtwy_Control/Inputs/Offset_Pot/Pot_Selection ==
  data: state_System input uint8; select_Position output uint8; enable_Normal output uint8; enable_CAN output uint8; CD_OFFSET_POT_FUNCTION parameter uint8; CD_WORKGROUP_FUNCTION parameter uint8; NON_FUNCTIONAL parameter uint8; SYS_NORMAL parameter uint8; CD_AUX_FUNCTION parameter uint8; AUX_EMEA_CONTROLS parameter uint8
function [select_Position, enable_Normal, enable_CAN] = fcn(state_System, CD_OFFSET_POT_FUNCTION, CD_WORKGROUP_FUNCTION, NON_FUNCTIONAL, SYS_NORMAL, CD_AUX_FUNCTION, AUX_EMEA_CONTROLS)

aVarTruthTableCondition_1 = false;
aVarTruthTableCondition_2 = false;
aVarTruthTableCondition_3 = false;


% Offset NonFunctional

aVarTruthTableCondition_1 = logical(CD_OFFSET_POT_FUNCTION == NON_FUNCTIONAL);

% System State Normal

aVarTruthTableCondition_2 = logical(state_System == SYS_NORMAL);

% CAN NonFunctional

aVarTruthTableCondition_3 = logical(CD_WORKGROUP_FUNCTION == NON_FUNCTIONAL);

if (aVarTruthTableCondition_1)
    aFcnTruthTableAction_1();
elseif (aVarTruthTableCondition_2 && aVarTruthTableCondition_3)
    aFcnTruthTableAction_2();
elseif (aVarTruthTableCondition_2 && ~aVarTruthTableCondition_3)
    aFcnTruthTableAction_3();
else % Default
    aFcnTruthTableAction_1();
end

function aFcnTruthTableAction_1()

% Non Functional

enable_Normal = uint8(0);
enable_CAN = uint8(0);
select_Position = uint8(0);

function aFcnTruthTableAction_2()

% Analog Pot

enable_Normal = uint8(1);
enable_CAN = uint8(0);
select_Position = uint8(0);

function aFcnTruthTableAction_3()

% CAN Pot

enable_Normal = uint8(0);
enable_CAN = uint8(1);
select_Position = uint8(1);

== stateflow chart MX_Gtwy_Control/Inputs/Aux_Pot/CAN_Aux_Pot/Aux_Pot ==
  state Aux_Pot_State [parallel]
    settings: executionOrder=2
    state CAN_OK
      state NORMAL
        en:
        position_AuxPotIn = rm_AuxPot + offset_AuxPotCal;
        position_AuxPotOut = position_AuxPotIn;
        du:
        position_AuxPotIn = rm_AuxPot + offset_AuxPotCal;
        position_AuxPotOut = position_AuxPotIn;
        (history)
        state Neutral
          en:
          state_AuxPot = NEUTRAL;
        state Rod_Active
          en:
          state_AuxPot =...
          ROD_ACTIVE;
        state Base_Active
          en:
          state_AuxPot =...
          BASE_ACTIVE;
        (default) -> Neutral
        Neutral -> Base_Active [order 2] : [position_AuxPotIn < base_Threshold]
        Neutral -> Rod_Active [order 1] : [position_AuxPotIn > rod_Threshold]
        Rod_Active -> Neutral [order 2] : [position_AuxPotIn <= rod_Threshold]
        Base_Active -> Neutral [order 2] : [position_AuxPotIn >= base_Threshold]
        Rod_Active -> Base_Active [order 1] : [position_AuxPotIn <= rod_Threshold...
            |  && position_AuxPotIn < base_Threshold]
        Base_Active -> Rod_Active [order 1] : [position_AuxPotIn >= rod_Threshold...
            |  && position_AuxPotIn > base_Threshold]
      state NOT_IN_NEUTRAL
        en:
        state_AuxPot = NOT_IN_NEUTRAL;
        position_AuxPotOut =...
        CD_AUXPOT_CENTER_RATIO;
      (default) -> NORMAL
      NORMAL -> NOT_IN_NEUTRAL : [state_AuxPotCal == NOT_IN_NEUTRAL]
      NOT_IN_NEUTRAL -> NORMAL : [state_AuxPotCal != NOT_IN_NEUTRAL]
    state CAN_Fault
      en:
      state_AuxPot = NORMAL;
      position_AuxPotOut =...
      CD_AUXPOT_CENTER_RATIO;
    (default) -> CAN_OK : {
        | base_Threshold = CD_AUXPOT_CENTER_RATIO - CD_AUXPOT_DEADBAND;
        | rod_Threshold = CD_AUXPOT_CENTER_RATIO + CD_AUXPOT_DEADBAND;
        | }
    CAN_OK -> CAN_Fault : [msgAge_BJM2Right > 1000]
  state Aux_Pot_Neutral_Check [parallel]
    settings: executionOrder=1
    state Initialize
      en:
      state_AuxPotCal = NORMAL;
      offset_AuxPotCal = 0;
    state WaitForPress
    state Wait_For_New_Cal
    note: if we are already NOT_IN_NEUTRAL
        | be sure to not beep again
    note: set the triple beep here instead of in the fault code
        | to be sure other aux beeps don't override the fault code beep
    state Joystick_Powerup
    (default) -> Initialize
    Initialize -> WaitForPress : [after(ONE_SECOND, tick)]
    WaitForPress -> (junction 4558) [order 1] : [(aux_Flow == AUX_FLOW_LOW || aux_Flow == AUX_FLOW_MEDIUM || aux_Flow == AUX_FLOW_STANDARD || aux_Flow == AUX_FLOW_HIGH)...
        | && state_Console == ACTIVE...
        | && state_Engine != ENGINE_CRANKING...
        | && msgAge_BJM2Right < 100]
    (junction 4558) -> (junction 4559)
    (junction 4559) -> (junction 4560) [order 1] : [rm_AuxPot >= CD_AUXPOT_CAL_DEADBAND_LOW...
        |  && rm_AuxPot <= CD_AUXPOT_CAL_DEADBAND_HIGH]
    (junction 4563) -> (junction 4558)
    (junction 4559) -> (junction 4561) [order 2]
    (junction 4560) -> (junction 4564) : {
        | state_AuxPotCal = NORMAL;
        | offset_AuxPotCal = int16(CD_AUXPOT_CENTER_RATIO) - rm_AuxPot;
        | }
    (junction 4561) -> (junction 4562) [order 1] : [state_AuxPot != NOT_IN_NEUTRAL]
    (junction 4561) -> (junction 4565) [order 2] : {
        | state_Buzzer = BEEP_NONE;
        | }
    (junction 4562) -> (junction 4566) : {
        | state_AuxPotCal = NOT_IN_NEUTRAL;
        | offset_AuxPotCal = 0;
        | state_Buzzer = TRIPLE_SHORT_BEEP;
        | }
    WaitForPress -> (junction 4563) [order 2] : [(aux_DebounceButton || aux_DetentHold)...
        | && (state_AuxEnable == INACTIVE || aux_Flow == AUX_FLOW_INACTIVE)...
        | && msgAge_BJM2Right < 100]
    (junction 4567) -> (junction 4563)
    (junction 4564) -> Wait_For_New_Cal
    (junction 4565) -> (junction 4566)
    (junction 4566) -> Wait_For_New_Cal
    Wait_For_New_Cal -> (junction 4567) [order 2] : [(aux_DebounceButton || aux_DetentHold)...
        | && (state_AuxEnable == INACTIVE || aux_Flow == AUX_FLOW_INACTIVE)]
    Wait_For_New_Cal -> WaitForPress [order 1] : [state_Console != ACTIVE]
    Joystick_Powerup -> WaitForPress : [after(ONE_SECOND, tick)]
    Wait_For_New_Cal -> Joystick_Powerup [order 3] : [state_Engine == ENGINE_CRANKING]
  data: aux_DebounceButton input uint8; aux_Flow input uint8; state_AuxEnable input uint8; aux_DetentHold input uint8; state_AuxPot output uint8; position_AuxPotOut output int16; position_AuxPotIn local int16 size=1; offset_AuxPotCal local int16; state_AuxPotCal local uint8; CD_AUXPOT_CENTER_RATIO parameter uint16; CD_AUXPOT_CAL_DEADBAND_HIGH parameter uint16; CD_AUXPOT_CAL_DEADBAND_LOW parameter uint16; NOT_IN_NEUTRAL parameter uint8; BASE_ACTIVE parameter uint8; base_Threshold local uint16; CD_AUXPOT_DEADBAND parameter uint8; NEUTRAL parameter uint8; ROD_ACTIVE parameter uint8; rod_Threshold local uint16; NORMAL parameter uint8; INACTIVE parameter uint8; ACTIVE parameter uint8; state_Console input uint8; state_Engine input uint8; msgAge_BJM2Right input uint16; rm_AuxPot input int16; ENGINE_CRANKING parameter uint8; TRIPLE_SHORT_BEEP parameter uint8; state_Buzzer data_store_memory double; BEEP_NONE parameter uint8; ONE_SECOND constant uint8 =100; AUX_FLOW_INACTIVE parameter uint8; AUX_FLOW_LOW parameter uint8; AUX_FLOW_STANDARD parameter uint8; AUX_FLOW_HIGH parameter uint8; AUX_FLOW_MEDIUM parameter uint8

== matlab function MX_Gtwy_Control/Inputs/Aux_Pot/Pot_Selection ==
  data: state_System input uint8; select_Position output uint8; enable_Normal output uint8; enable_CAN output uint8; CD_AUXPOT_FUNCTION parameter uint8; CD_WORKGROUP_FUNCTION parameter uint8; NON_FUNCTIONAL parameter uint8; SYS_NORMAL parameter uint8; AUX_NON_FUNCTIONAL parameter uint8; AUX_EMEA_CONTROLS parameter uint8
function [select_Position, enable_Normal, enable_CAN] = fcn(state_System, CD_AUXPOT_FUNCTION, CD_WORKGROUP_FUNCTION, NON_FUNCTIONAL, SYS_NORMAL, AUX_NON_FUNCTIONAL, AUX_EMEA_CONTROLS)

aVarTruthTableCondition_1 = false;
aVarTruthTableCondition_2 = false;
aVarTruthTableCondition_3 = false;


% Aux NonFunctional

aVarTruthTableCondition_1 = logical(CD_AUXPOT_FUNCTION == NON_FUNCTIONAL);

% System State Normal

aVarTruthTableCondition_2 = logical(state_System == SYS_NORMAL);

% CAN NonFunctional

aVarTruthTableCondition_3 = logical(CD_WORKGROUP_FUNCTION == NON_FUNCTIONAL);

if (aVarTruthTableCondition_1)
    aFcnTruthTableAction_1();
elseif (aVarTruthTableCondition_2 && aVarTruthTableCondition_3)
    aFcnTruthTableAction_2();
elseif (aVarTruthTableCondition_2 && ~aVarTruthTableCondition_3)
    aFcnTruthTableAction_3();
else % Default
    aFcnTruthTableAction_1();
end

function aFcnTruthTableAction_1()

% Non Functional

enable_Normal =  uint8(0);
enable_CAN =  uint8(0);
select_Position = uint8(0);

function aFcnTruthTableAction_2()

% Analog Pot

enable_Normal = uint8(1);
enable_CAN = uint8(0);
select_Position = uint8(0);

function aFcnTruthTableAction_3()

% CAN Pot

enable_Normal =   uint8(0);
enable_CAN =  uint8(1);
select_Position = uint8(1);

== stateflow chart MX_Gtwy_Control/Inputs/Aux_Button/AuxButtonLogic/Aux_Pot ==
  state INACTIVE
    en:
    aux_DebounceButton = INACTIVE;
    aux_DetentHold = INACTIVE;
  state INACTIVE_WAIT
  state DETENT
    en:
    aux_DetentHold = ACTIVE;
    du:
    aux_DetentHold = INACTIVE;
  state AUX_FALLING_EDGE
    en:aux_DebounceButton = ACTIVE;
  state HOLD
  (default) -> INACTIVE
  INACTIVE -> INACTIVE_WAIT [order 2] : [aux_Button...
      | && (state_Buttons != NORMAL)]
  INACTIVE_WAIT -> INACTIVE : [!aux_Button]
  DETENT -> INACTIVE : [!aux_Button]
  AUX_FALLING_EDGE -> INACTIVE
  INACTIVE -> HOLD [order 1] : [aux_Button...
      | && (state_Buttons == NORMAL)...
      | && (state_Console == ACTIVE)]
  HOLD -> AUX_FALLING_EDGE [order 2] : [!aux_Button]
  HOLD -> DETENT [order 1] : [after(CD_AUX_DETENT_HOLD_TIME,tick)]
  data: aux_DebounceButton output uint8; aux_Button input boolean; NORMAL parameter uint8; INACTIVE parameter uint8; state_Buttons input uint8; ACTIVE parameter uint8; state_Console input uint8; aux_DetentHold output uint8; CD_AUX_DETENT_HOLD_TIME parameter uint16

== stateflow chart MX_Gtwy_Control/System/EMEA Control Mode/Left Handle Switch ==
  state Default_InactiveCheck
    en:
    request = 0;
  state DebounceActive
  state Inactive
    en:
    request = 0;
  state Active
    en:
    request = 1;
    du:
    request = 0;
  (default) -> Default_InactiveCheck
  Default_InactiveCheck -> Inactive : [din_Sw == INACTIVE]
  Inactive -> DebounceActive : [din_Sw == ACTIVE]
  DebounceActive -> Inactive [order 2] : [din_Sw == INACTIVE]
  Active -> Inactive : [din_Sw == INACTIVE]
  DebounceActive -> Active [order 1] : [after(CD_EMEA_CONTROLS_HOLD_TIME,tick)]
  data: din_Sw input boolean; INACTIVE parameter uint8; ACTIVE parameter uint8; request output boolean; CD_EMEA_CONTROLS_HOLD_TIME parameter uint16
